simulink model "vegas01_4tap_1024ch_220_r6b"  (Simulink 7.6)
settings: Solver=ode45  StartTime=0.0  StopTime=128000  RelTol=1e-3
undefined symbols (not defined in the file): pfb_coeff_gen_calc, ROACH
libraries: xbsIndex_r4 x12404, xps_library x53, xbsReplacements_r4 x42, casper_library_misc x38, casper_library_delays x32, dspsigops x16, simulink x8
(part 3 of 88)

== system vegas01_4tap_1024ch_220_r6b/cram4 ==
  In1 : Inport
  In2 : Inport  Port=2
  In3 : Inport  Port=3
  In4 : Inport  Port=4
  In5 : Inport  Port=5
  In6 : Inport  Port=6
  In7 : Inport  Port=7
  In8 : Inport  Port=8
  In9 : Inport  Port=9
  In10 : Inport  Port=10
  In11 : Inport  Port=11
  In12 : Inport  Port=12
  In13 : Inport  Port=13
  In14 : Inport  Port=14
  In15 : Inport  Port=15
  In16 : Inport  Port=16
  Concat : xbsIndex_r4/Concat  num_inputs=16  dbl_ovrd=off  block_type=concat
      settings: block_version=10.1.2
  Reinterp{1..16} (16 alike) : xbsIndex_r4/Reinterpret  force_arith_type=on  arith_type=Unsigned  force_bin_pt=on  bin_pt=0  block_type=reinterpret
      Reinterp1 settings: infoedit="Changes signal type without altering the binary representation.   You can changed the signal between signed and unsigned, and relocate the binary point.<P><P>Hardware notes: In hardware this block costs nothing.<P><P>Example:  Suppose the input is 6 bits wide, signed, with 2 fractional bits, and the output is forced to unsigned with 0 fractional bits.  Then an input of -2.0 (1110.00 in binary 2's complement) becomes an output of 56 (111000 in binary)."  block_version=10.1.2
      Reinterp10 settings: infoedit="Changes signal type without altering the binary representation.   You can changed the signal between signed and unsigned, and relocate the binary point.<br><br>Hardware notes: In hardware this block costs nothing.<br><br>Example:  Suppose the input is 6 bits wide, signed, with 2 fractional bits, and the output is forced to unsigned with 0 fractional bits.  Then an input of -2.0 (1110.00 in binary 2's complement) becomes an output of 56 (111000 in binary)."
      Reinterp11 settings: infoedit="Changes signal type without altering the binary representation.   You can changed the signal between signed and unsigned, and relocate the binary point.<br><br>Hardware notes: In hardware this block costs nothing.<br><br>Example:  Suppose the input is 6 bits wide, signed, with 2 fractional bits, and the output is forced to unsigned with 0 fractional bits.  Then an input of -2.0 (1110.00 in binary 2's complement) becomes an output of 56 (111000 in binary)."
      Reinterp12 settings: infoedit="Changes signal type without altering the binary representation.   You can changed the signal between signed and unsigned, and relocate the binary point.<br><br>Hardware notes: In hardware this block costs nothing.<br><br>Example:  Suppose the input is 6 bits wide, signed, with 2 fractional bits, and the output is forced to unsigned with 0 fractional bits.  Then an input of -2.0 (1110.00 in binary 2's complement) becomes an output of 56 (111000 in binary)."
      Reinterp13 settings: infoedit="Changes signal type without altering the binary representation.   You can changed the signal between signed and unsigned, and relocate the binary point.<br><br>Hardware notes: In hardware this block costs nothing.<br><br>Example:  Suppose the input is 6 bits wide, signed, with 2 fractional bits, and the output is forced to unsigned with 0 fractional bits.  Then an input of -2.0 (1110.00 in binary 2's complement) becomes an output of 56 (111000 in binary)."
      Reinterp14 settings: infoedit="Changes signal type without altering the binary representation.   You can changed the signal between signed and unsigned, and relocate the binary point.<br><br>Hardware notes: In hardware this block costs nothing.<br><br>Example:  Suppose the input is 6 bits wide, signed, with 2 fractional bits, and the output is forced to unsigned with 0 fractional bits.  Then an input of -2.0 (1110.00 in binary 2's complement) becomes an output of 56 (111000 in binary)."
      Reinterp15 settings: infoedit="Changes signal type without altering the binary representation.   You can changed the signal between signed and unsigned, and relocate the binary point.<br><br>Hardware notes: In hardware this block costs nothing.<br><br>Example:  Suppose the input is 6 bits wide, signed, with 2 fractional bits, and the output is forced to unsigned with 0 fractional bits.  Then an input of -2.0 (1110.00 in binary 2's complement) becomes an output of 56 (111000 in binary)."
      Reinterp16 settings: infoedit="Changes signal type without altering the binary representation.   You can changed the signal between signed and unsigned, and relocate the binary point.<br><br>Hardware notes: In hardware this block costs nothing.<br><br>Example:  Suppose the input is 6 bits wide, signed, with 2 fractional bits, and the output is forced to unsigned with 0 fractional bits.  Then an input of -2.0 (1110.00 in binary 2's complement) becomes an output of 56 (111000 in binary)."
      Reinterp2 settings: infoedit="Changes signal type without altering the binary representation.   You can changed the signal between signed and unsigned, and relocate the binary point.<P><P>Hardware notes: In hardware this block costs nothing.<P><P>Example:  Suppose the input is 6 bits wide, signed, with 2 fractional bits, and the output is forced to unsigned with 0 fractional bits.  Then an input of -2.0 (1110.00 in binary 2's complement) becomes an output of 56 (111000 in binary)."  block_version=10.1.2
      Reinterp3 settings: infoedit="Changes signal type without altering the binary representation.   You can changed the signal between signed and unsigned, and relocate the binary point.<br><br>Hardware notes: In hardware this block costs nothing.<br><br>Example:  Suppose the input is 6 bits wide, signed, with 2 fractional bits, and the output is forced to unsigned with 0 fractional bits.  Then an input of -2.0 (1110.00 in binary 2's complement) becomes an output of 56 (111000 in binary)."
      Reinterp4 settings: infoedit="Changes signal type without altering the binary representation.   You can changed the signal between signed and unsigned, and relocate the binary point.<br><br>Hardware notes: In hardware this block costs nothing.<br><br>Example:  Suppose the input is 6 bits wide, signed, with 2 fractional bits, and the output is forced to unsigned with 0 fractional bits.  Then an input of -2.0 (1110.00 in binary 2's complement) becomes an output of 56 (111000 in binary)."
      Reinterp5 settings: infoedit="Changes signal type without altering the binary representation.   You can changed the signal between signed and unsigned, and relocate the binary point.<br><br>Hardware notes: In hardware this block costs nothing.<br><br>Example:  Suppose the input is 6 bits wide, signed, with 2 fractional bits, and the output is forced to unsigned with 0 fractional bits.  Then an input of -2.0 (1110.00 in binary 2's complement) becomes an output of 56 (111000 in binary)."
      Reinterp6 settings: infoedit="Changes signal type without altering the binary representation.   You can changed the signal between signed and unsigned, and relocate the binary point.<br><br>Hardware notes: In hardware this block costs nothing.<br><br>Example:  Suppose the input is 6 bits wide, signed, with 2 fractional bits, and the output is forced to unsigned with 0 fractional bits.  Then an input of -2.0 (1110.00 in binary 2's complement) becomes an output of 56 (111000 in binary)."
      Reinterp7 settings: infoedit="Changes signal type without altering the binary representation.   You can changed the signal between signed and unsigned, and relocate the binary point.<br><br>Hardware notes: In hardware this block costs nothing.<br><br>Example:  Suppose the input is 6 bits wide, signed, with 2 fractional bits, and the output is forced to unsigned with 0 fractional bits.  Then an input of -2.0 (1110.00 in binary 2's complement) becomes an output of 56 (111000 in binary)."
      Reinterp8 settings: infoedit="Changes signal type without altering the binary representation.   You can changed the signal between signed and unsigned, and relocate the binary point.<br><br>Hardware notes: In hardware this block costs nothing.<br><br>Example:  Suppose the input is 6 bits wide, signed, with 2 fractional bits, and the output is forced to unsigned with 0 fractional bits.  Then an input of -2.0 (1110.00 in binary 2's complement) becomes an output of 56 (111000 in binary)."
      Reinterp9 settings: infoedit="Changes signal type without altering the binary representation.   You can changed the signal between signed and unsigned, and relocate the binary point.<br><br>Hardware notes: In hardware this block costs nothing.<br><br>Example:  Suppose the input is 6 bits wide, signed, with 2 fractional bits, and the output is forced to unsigned with 0 fractional bits.  Then an input of -2.0 (1110.00 in binary 2's complement) becomes an output of 56 (111000 in binary)."
  Out : Outport
  wires:
  Concat/1 -> Out/1
  In1/1 -> Reinterp1/1
  Reinterp1/1 -> Concat/1
  In2/1 -> Reinterp2/1
  Reinterp2/1 -> Concat/2
  In3/1 -> Reinterp3/1
  Reinterp3/1 -> Concat/3
  In4/1 -> Reinterp4/1
  Reinterp4/1 -> Concat/4
  In5/1 -> Reinterp5/1
  Reinterp5/1 -> Concat/5
  In6/1 -> Reinterp6/1
  Reinterp6/1 -> Concat/6
  In7/1 -> Reinterp7/1
  Reinterp7/1 -> Concat/7
  In8/1 -> Reinterp8/1
  Reinterp8/1 -> Concat/8
  In9/1 -> Reinterp9/1
  Reinterp9/1 -> Concat/9
  In10/1 -> Reinterp10/1
  Reinterp10/1 -> Concat/10
  In11/1 -> Reinterp11/1
  Reinterp11/1 -> Concat/11
  In12/1 -> Reinterp12/1
  Reinterp12/1 -> Concat/12
  In13/1 -> Reinterp13/1
  Reinterp13/1 -> Concat/13
  In14/1 -> Reinterp14/1
  Reinterp14/1 -> Concat/14
  In15/1 -> Reinterp15/1
  Reinterp15/1 -> Concat/15
  In16/1 -> Reinterp16/1
  Reinterp16/1 -> Concat/16

== system vegas01_4tap_1024ch_220_r6b/cram5 ==
  In1 : Inport
  In2 : Inport  Port=2
  Concat : xbsIndex_r4/Concat  num_inputs=2  dbl_ovrd=off  block_type=concat
      settings: block_version=10.1.2
  Reinterp1 : xbsIndex_r4/Reinterpret  force_arith_type=on  arith_type=Unsigned  force_bin_pt=on  bin_pt=0  block_type=reinterpret
      settings: infoedit="Changes signal type without altering the binary representation.   You can changed the signal between signed and unsigned, and relocate the binary point.<P><P>Hardware notes: In hardware this block costs nothing.<P><P>Example:  Suppose the input is 6 bits wide, signed, with 2 fractional bits, and the output is forced to unsigned with 0 fractional bits.  Then an input of -2.0 (1110.00 in binary 2's complement) becomes an output of 56 (111000 in binary)."  block_version=10.1.2
  Reinterp2 : xbsIndex_r4/Reinterpret  force_arith_type=on  arith_type=Unsigned  force_bin_pt=on  bin_pt=0  block_type=reinterpret
      settings: infoedit="Changes signal type without altering the binary representation.   You can changed the signal between signed and unsigned, and relocate the binary point.<P><P>Hardware notes: In hardware this block costs nothing.<P><P>Example:  Suppose the input is 6 bits wide, signed, with 2 fractional bits, and the output is forced to unsigned with 0 fractional bits.  Then an input of -2.0 (1110.00 in binary 2's complement) becomes an output of 56 (111000 in binary)."  block_version=10.1.2
  Out : Outport
  wires:
  Reinterp2/1 -> Concat/2
  In2/1 -> Reinterp2/1
  Reinterp1/1 -> Concat/1
  In1/1 -> Reinterp1/1
  Concat/1 -> Out/1

== system vegas01_4tap_1024ch_220_r6b/fft_wideband_real1 ==
  sync : Inport
  shift : Inport  Port=2
  din_0 : Inport  Port=3
  din_1 : Inport  Port=4
  din_2 : Inport  Port=5
  din_3 : Inport  Port=6
  din_4 : Inport  Port=7
  din_5 : Inport  Port=8
  din_6 : Inport  Port=9
  din_7 : Inport  Port=10
  fft_biplex_real_4x0 : Subsystem
      settings: AttributesFormatString=|
      | Virtex5
      | 8 stages
      | [18,18]
      | Truncate
      | Wrap
      | 0 0 0 0 0 0 0 0 0 0 0 0 0 0 0 0 0 0 0 0
  fft_biplex_real_4x1 : Subsystem
      settings: AttributesFormatString=|
      | Virtex5
      | 8 stages
      | [18,18]
      | Truncate
      | Wrap
      | 0 0 0 0 0 0 0 0 0 0 0 0 0 0 0 0 0 0 0 0
  fft_direct : Subsystem
      settings: AttributesFormatString=|
      | Virtex5
      | stages [9  10  11] of 11
      | [18,18]
      | Truncate
      | Wrap
      | 0 0 0 0 0 0 0
  fft_unscrambler : Subsystem
      settings: AttributesFormatString=FFTSize=10, n_inputs=2
  in_del_4x0pol1 : Subsystem
      settings: AttributesFormatString=Latency = 0
  in_del_4x0pol2 : Subsystem
      settings: AttributesFormatString=Latency = 0
  in_del_4x0pol3 : Subsystem
      settings: AttributesFormatString=Latency = 0
  in_del_4x0pol4 : Subsystem
      settings: AttributesFormatString=Latency = 0
  in_del_4x1pol1 : Subsystem
      settings: AttributesFormatString=Latency = 0
  in_del_4x1pol2 : Subsystem
      settings: AttributesFormatString=Latency = 0
  in_del_4x1pol3 : Subsystem
      settings: AttributesFormatString=Latency = 0
  in_del_4x1pol4 : Subsystem
      settings: AttributesFormatString=Latency = 0
  in_del_sync_4x0 : Subsystem
      settings: AttributesFormatString=Latency = 0
  in_del_sync_4x1 : Subsystem
      settings: AttributesFormatString=Latency = 0
  of_det : xbsIndex_r4/Logical  logical_function=OR  inputs=3  en=off  latency=1  precision=Full  arith_type=Unsigned  n_bits=16  bin_pt=0  align_bp=on  dbl_ovrd=off  block_type=logical
  out_del_4x0pol1 : Subsystem
      settings: AttributesFormatString=Latency = 0
  out_del_4x0pol2 : Subsystem
      settings: AttributesFormatString=Latency = 0
  out_del_4x0pol3 : Subsystem
      settings: AttributesFormatString=Latency = 0
  out_del_4x0pol4 : Subsystem
      settings: AttributesFormatString=Latency = 0
  out_del_4x1pol1 : Subsystem
      settings: AttributesFormatString=Latency = 0
  out_del_4x1pol2 : Subsystem
      settings: AttributesFormatString=Latency = 0
  out_del_4x1pol3 : Subsystem
      settings: AttributesFormatString=Latency = 0
  out_del_4x1pol4 : Subsystem
      settings: AttributesFormatString=Latency = 0
  out_del_sync_4x0 : Subsystem
      settings: AttributesFormatString=Latency = 0
  shift_slice : xbsIndex_r4/Slice  nbits=3  boolean_output=off  mode=Lower Bit Location + Width  bit1=0  base1=MSB of Input  bit0=8  base0=LSB of Input  dbl_ovrd=off  block_type=slice
  sync_out : Outport
  dout0 : Outport  Port=2
  dout1 : Outport  Port=3
  dout2 : Outport  Port=4
  dout3 : Outport  Port=5
  of : Outport  Port=6
  wires:
  shift/1 -> fft_biplex_real_4x1/2(shift), fft_biplex_real_4x0/2(shift), shift_slice/1
  sync/1 -> in_del_sync_4x1/1(d), in_del_sync_4x0/1(d)
  of_det/1 -> of/1
  fft_direct/10(of) -> of_det/3
  fft_biplex_real_4x1/6(of) -> of_det/2
  fft_biplex_real_4x0/6(of) -> of_det/1
  fft_unscrambler/5(Out4) -> dout3/1
  fft_unscrambler/4(Out3) -> dout2/1
  fft_unscrambler/3(Out2) -> dout1/1
  fft_unscrambler/2(Out1) -> dout0/1
  fft_unscrambler/1(sync_out) -> sync_out/1
  fft_direct/5(dout_3) -> fft_unscrambler/5(In4)
  fft_direct/4(dout_2) -> fft_unscrambler/4(In3)
  fft_direct/3(dout_1) -> fft_unscrambler/3(In2)
  fft_direct/2(dout_0) -> fft_unscrambler/2(In1)
  fft_direct/1(sync_out) -> fft_unscrambler/1(sync)
  out_del_4x1pol4/1(q) -> fft_direct/10(din_7)
  out_del_4x1pol3/1(q) -> fft_direct/9(din_6)
  out_del_4x1pol2/1(q) -> fft_direct/8(din_5)
  out_del_4x1pol1/1(q) -> fft_direct/7(din_4)
  out_del_4x0pol4/1(q) -> fft_direct/6(din_3)
  out_del_4x0pol3/1(q) -> fft_direct/5(din_2)
  out_del_4x0pol2/1(q) -> fft_direct/4(din_1)
  out_del_4x0pol1/1(q) -> fft_direct/3(din_0)
  shift_slice/1 -> fft_direct/2(shift)
  out_del_sync_4x0/1(q) -> fft_direct/1(sync)
  fft_biplex_real_4x1/5(pol4_out) -> out_del_4x1pol4/1(d)
  fft_biplex_real_4x1/4(pol3_out) -> out_del_4x1pol3/1(d)
  fft_biplex_real_4x1/3(pol2_out) -> out_del_4x1pol2/1(d)
  fft_biplex_real_4x1/2(pol1_out) -> out_del_4x1pol1/1(d)
  in_del_4x1pol4/1(q) -> fft_biplex_real_4x1/6(pol4)
  in_del_4x1pol3/1(q) -> fft_biplex_real_4x1/5(pol3)
  in_del_4x1pol2/1(q) -> fft_biplex_real_4x1/4(pol2)
  in_del_4x1pol1/1(q) -> fft_biplex_real_4x1/3(pol1)
  in_del_sync_4x1/1(q) -> fft_biplex_real_4x1/1(sync)
  din_7/1 -> in_del_4x1pol4/1(d)
  din_6/1 -> in_del_4x1pol3/1(d)
  din_5/1 -> in_del_4x1pol2/1(d)
  din_4/1 -> in_del_4x1pol1/1(d)
  fft_biplex_real_4x0/1(sync_out) -> out_del_sync_4x0/1(d)
  fft_biplex_real_4x0/5(pol4_out) -> out_del_4x0pol4/1(d)
  fft_biplex_real_4x0/4(pol3_out) -> out_del_4x0pol3/1(d)
  fft_biplex_real_4x0/3(pol2_out) -> out_del_4x0pol2/1(d)
  fft_biplex_real_4x0/2(pol1_out) -> out_del_4x0pol1/1(d)
  in_del_4x0pol4/1(q) -> fft_biplex_real_4x0/6(pol4)
  in_del_4x0pol3/1(q) -> fft_biplex_real_4x0/5(pol3)
  in_del_4x0pol2/1(q) -> fft_biplex_real_4x0/4(pol2)
  in_del_4x0pol1/1(q) -> fft_biplex_real_4x0/3(pol1)
  in_del_sync_4x0/1(q) -> fft_biplex_real_4x0/1(sync)
  din_3/1 -> in_del_4x0pol4/1(d)
  din_2/1 -> in_del_4x0pol3/1(d)
  din_1/1 -> in_del_4x0pol2/1(d)
  din_0/1 -> in_del_4x0pol1/1(d)

== system vegas01_4tap_1024ch_220_r6b/fft_wideband_real4 ==
  sync : Inport
  shift : Inport  Port=2
  din_0 : Inport  Port=3
  din_1 : Inport  Port=4
  din_2 : Inport  Port=5
  din_3 : Inport  Port=6
  din_4 : Inport  Port=7
  din_5 : Inport  Port=8
  din_6 : Inport  Port=9
  din_7 : Inport  Port=10
  fft_biplex_real_4x0 : Subsystem
      settings: AttributesFormatString=|
      | Virtex5
      | 8 stages
      | [18,18]
      | Truncate
      | Wrap
      | 0 0 0 0 0 0 0 0 0 0 0 0 0 0 0 0 0 0 0 0
  fft_biplex_real_4x1 : Subsystem
      settings: AttributesFormatString=|
      | Virtex5
      | 8 stages
      | [18,18]
      | Truncate
      | Wrap
      | 0 0 0 0 0 0 0 0 0 0 0 0 0 0 0 0 0 0 0 0
  fft_direct : Subsystem
      settings: AttributesFormatString=|
      | Virtex5
      | stages [9  10  11] of 11
      | [18,18]
      | Truncate
      | Wrap
      | 0 0 0 0 0 0 0
  fft_unscrambler : Subsystem
      settings: AttributesFormatString=FFTSize=10, n_inputs=2
  in_del_4x0pol1 : Subsystem
      settings: AttributesFormatString=Latency = 0
  in_del_4x0pol2 : Subsystem
      settings: AttributesFormatString=Latency = 0
  in_del_4x0pol3 : Subsystem
      settings: AttributesFormatString=Latency = 0
  in_del_4x0pol4 : Subsystem
      settings: AttributesFormatString=Latency = 0
  in_del_4x1pol1 : Subsystem
      settings: AttributesFormatString=Latency = 0
  in_del_4x1pol2 : Subsystem
      settings: AttributesFormatString=Latency = 0
  in_del_4x1pol3 : Subsystem
      settings: AttributesFormatString=Latency = 0
  in_del_4x1pol4 : Subsystem
      settings: AttributesFormatString=Latency = 0
  in_del_sync_4x0 : Subsystem
      settings: AttributesFormatString=Latency = 0
  in_del_sync_4x1 : Subsystem
      settings: AttributesFormatString=Latency = 0
  of_det : xbsIndex_r4/Logical  logical_function=OR  inputs=3  en=off  latency=1  precision=Full  arith_type=Unsigned  n_bits=16  bin_pt=0  align_bp=on  dbl_ovrd=off  block_type=logical
  out_del_4x0pol1 : Subsystem
      settings: AttributesFormatString=Latency = 0
  out_del_4x0pol2 : Subsystem
      settings: AttributesFormatString=Latency = 0
  out_del_4x0pol3 : Subsystem
      settings: AttributesFormatString=Latency = 0
  out_del_4x0pol4 : Subsystem
      settings: AttributesFormatString=Latency = 0
  out_del_4x1pol1 : Subsystem
      settings: AttributesFormatString=Latency = 0
  out_del_4x1pol2 : Subsystem
      settings: AttributesFormatString=Latency = 0
  out_del_4x1pol3 : Subsystem
      settings: AttributesFormatString=Latency = 0
  out_del_4x1pol4 : Subsystem
      settings: AttributesFormatString=Latency = 0
  out_del_sync_4x0 : Subsystem
      settings: AttributesFormatString=Latency = 0
  shift_slice : xbsIndex_r4/Slice  nbits=3  boolean_output=off  mode=Lower Bit Location + Width  bit1=0  base1=MSB of Input  bit0=8  base0=LSB of Input  dbl_ovrd=off  block_type=slice
  sync_out : Outport
  dout0 : Outport  Port=2
  dout1 : Outport  Port=3
  dout2 : Outport  Port=4
  dout3 : Outport  Port=5
  of : Outport  Port=6
  wires:
  shift/1 -> fft_biplex_real_4x1/2(shift), fft_biplex_real_4x0/2(shift), shift_slice/1
  sync/1 -> in_del_sync_4x1/1(d), in_del_sync_4x0/1(d)
  of_det/1 -> of/1
  fft_direct/10(of) -> of_det/3
  fft_biplex_real_4x1/6(of) -> of_det/2
  fft_biplex_real_4x0/6(of) -> of_det/1
  fft_unscrambler/5(Out4) -> dout3/1
  fft_unscrambler/4(Out3) -> dout2/1
  fft_unscrambler/3(Out2) -> dout1/1
  fft_unscrambler/2(Out1) -> dout0/1
  fft_unscrambler/1(sync_out) -> sync_out/1
  fft_direct/5(dout_3) -> fft_unscrambler/5(In4)
  fft_direct/4(dout_2) -> fft_unscrambler/4(In3)
  fft_direct/3(dout_1) -> fft_unscrambler/3(In2)
  fft_direct/2(dout_0) -> fft_unscrambler/2(In1)
  fft_direct/1(sync_out) -> fft_unscrambler/1(sync)
  out_del_4x1pol4/1(q) -> fft_direct/10(din_7)
  out_del_4x1pol3/1(q) -> fft_direct/9(din_6)
  out_del_4x1pol2/1(q) -> fft_direct/8(din_5)
  out_del_4x1pol1/1(q) -> fft_direct/7(din_4)
  out_del_4x0pol4/1(q) -> fft_direct/6(din_3)
  out_del_4x0pol3/1(q) -> fft_direct/5(din_2)
  out_del_4x0pol2/1(q) -> fft_direct/4(din_1)
  out_del_4x0pol1/1(q) -> fft_direct/3(din_0)
  shift_slice/1 -> fft_direct/2(shift)
  out_del_sync_4x0/1(q) -> fft_direct/1(sync)
  fft_biplex_real_4x1/5(pol4_out) -> out_del_4x1pol4/1(d)
  fft_biplex_real_4x1/4(pol3_out) -> out_del_4x1pol3/1(d)
  fft_biplex_real_4x1/3(pol2_out) -> out_del_4x1pol2/1(d)
  fft_biplex_real_4x1/2(pol1_out) -> out_del_4x1pol1/1(d)
  in_del_4x1pol4/1(q) -> fft_biplex_real_4x1/6(pol4)
  in_del_4x1pol3/1(q) -> fft_biplex_real_4x1/5(pol3)
  in_del_4x1pol2/1(q) -> fft_biplex_real_4x1/4(pol2)
  in_del_4x1pol1/1(q) -> fft_biplex_real_4x1/3(pol1)
  in_del_sync_4x1/1(q) -> fft_biplex_real_4x1/1(sync)
  din_7/1 -> in_del_4x1pol4/1(d)
  din_6/1 -> in_del_4x1pol3/1(d)
  din_5/1 -> in_del_4x1pol2/1(d)
  din_4/1 -> in_del_4x1pol1/1(d)
  fft_biplex_real_4x0/1(sync_out) -> out_del_sync_4x0/1(d)
  fft_biplex_real_4x0/5(pol4_out) -> out_del_4x0pol4/1(d)
  fft_biplex_real_4x0/4(pol3_out) -> out_del_4x0pol3/1(d)
  fft_biplex_real_4x0/3(pol2_out) -> out_del_4x0pol2/1(d)
  fft_biplex_real_4x0/2(pol1_out) -> out_del_4x0pol1/1(d)
  in_del_4x0pol4/1(q) -> fft_biplex_real_4x0/6(pol4)
  in_del_4x0pol3/1(q) -> fft_biplex_real_4x0/5(pol3)
  in_del_4x0pol2/1(q) -> fft_biplex_real_4x0/4(pol2)
  in_del_4x0pol1/1(q) -> fft_biplex_real_4x0/3(pol1)
  in_del_sync_4x0/1(q) -> fft_biplex_real_4x0/1(sync)
  din_3/1 -> in_del_4x0pol4/1(d)
  din_2/1 -> in_del_4x0pol3/1(d)
  din_1/1 -> in_del_4x0pol2/1(d)
  din_0/1 -> in_del_4x0pol1/1(d)
